simulink library "Garbage"  (R2023a)
settings: Solver=ode45  StartTime=0.0  StopTime=10.0  RelTol=1e-3  EnableMultiTasking=on
undefined symbols (not defined in the file or in its folder): c, ending_column_index, ending_row_index, framebuffer_16x1, framebuffer_2x2, framebuffer_4x4, framebuffer_8x8, HEIGHT, hot, POS_X, POS_Y, starting_column_index, starting_row_index, WIDTH
libraries: Memory_Blocks_Lib x459, simulink x235, simulink_extras x132, dspmtrx3 x116, Bit_Multiplexers_Lib x67, Bit_Demultiplexers_Lib x49, Byte_Multiplexers_Lib x40, Miscellaneous x38, Computer_Library x17, Decoders_Lib x14, commutil2 x12, Arithmetic_Operations_Lib x8
(part 9 of 26)

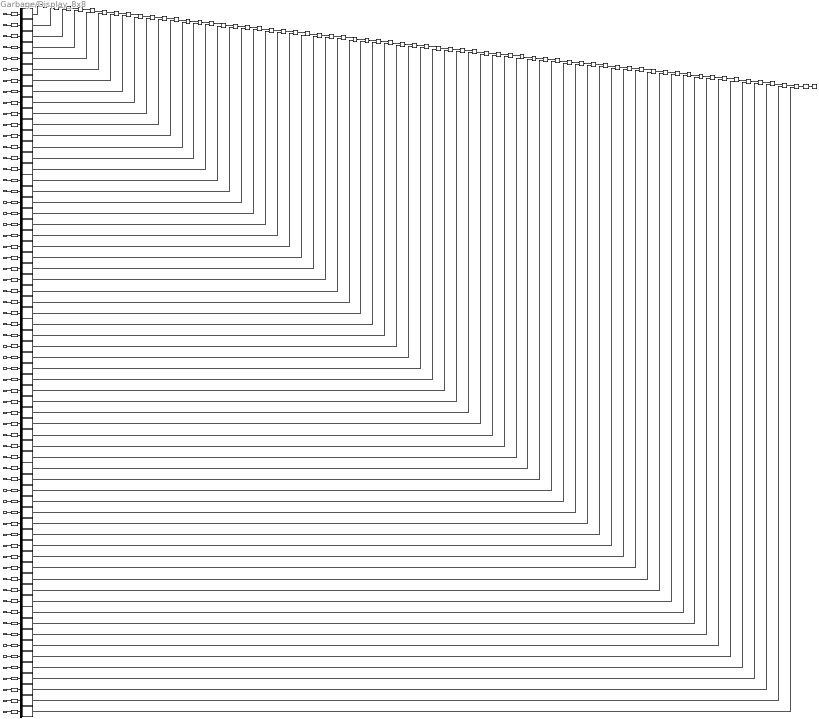
== system Garbage/Display_8x8 ==
  Row1_Col1(8x1B) : Inport
  Row1_Col2(8x1B) : Inport  Port=2
  Row1_Col3(8x1B) : Inport  Port=3
  Row1_Col4(8x1B) : Inport  Port=4
  Row1_Col5(8x1B) : Inport  Port=5
  Row1_Col6(8x1B) : Inport  Port=6
  Row1_Col7(8x1B) : Inport  Port=7
  Row1_Col8(8x1B) : Inport  Port=8
  Row2_Col1(8x1B) : Inport  Port=9
  Row2_Col2(8x1B) : Inport  Port=10
  Row2_Col3(8x1B) : Inport  Port=11
  Row2_Col4(8x1B) : Inport  Port=12
  Row2_Col5(8x1B) : Inport  Port=13
  Row2_Col6(8x1B) : Inport  Port=14
  Row2_Col7(8x1B) : Inport  Port=15
  Row2_Col8(8x1B) : Inport  Port=16
  Row3_Col1(8x1B) : Inport  Port=17
  Row3_Col2(8x1B) : Inport  Port=18
  Row3_Col3(8x1B) : Inport  Port=19
  Row3_Col4(8x1B) : Inport  Port=20
  Row3_Col5(8x1B) : Inport  Port=21
  Row3_Col6(8x1B) : Inport  Port=22
  Row3_Col7(8x1B) : Inport  Port=23
  Row3_Col8(8x1B) : Inport  Port=24
  Row4_Col1(8x1B) : Inport  Port=25
  Row4_Col2(8x1B) : Inport  Port=26
  Row4_Col3(8x1B) : Inport  Port=27
  Row4_Col4(8x1B) : Inport  Port=28
  Row4_Col5(8x1B) : Inport  Port=29
  Row4_Col6(8x1B) : Inport  Port=30
  Row4_Col7(8x1B) : Inport  Port=31
  Row4_Col8(8x1B) : Inport  Port=32
  Row5_Col1(8x1B) : Inport  Port=33
  Row5_Col2(8x1B) : Inport  Port=34
  Row5_Col3(8x1B) : Inport  Port=35
  Row5_Col4(8x1B) : Inport  Port=36
  Row5_Col5(8x1B) : Inport  Port=37
  Row5_Col6(8x1B) : Inport  Port=38
  Row5_Col7(8x1B) : Inport  Port=39
  Row5_Col8(8x1B) : Inport  Port=40
  Row6_Col1(8x1B) : Inport  Port=41
  Row6_Col2(8x1B) : Inport  Port=42
  Row6_Col3(8x1B) : Inport  Port=43
  Row6_Col4(8x1B) : Inport  Port=44
  Row6_Col5(8x1B) : Inport  Port=45
  Row6_Col6(8x1B) : Inport  Port=46
  Row6_Col7(8x1B) : Inport  Port=47
  Row6_Col8(8x1B) : Inport  Port=48
  Row7_Col1(8x1B) : Inport  Port=49
  Row7_Col2(8x1B) : Inport  Port=50
  Row7_Col3(8x1B) : Inport  Port=51
  Row7_Col4(8x1B) : Inport  Port=52
  Row7_Col5(8x1B) : Inport  Port=53
  Row7_Col6(8x1B) : Inport  Port=54
  Row7_Col7(8x1B) : Inport  Port=55
  Row7_Col8(8x1B) : Inport  Port=56
  Row8_Col1(8x1B) : Inport  Port=57
  Row8_Col2(8x1B) : Inport  Port=58
  Row8_Col3(8x1B) : Inport  Port=59
  Row8_Col4(8x1B) : Inport  Port=60
  Row8_Col5(8x1B) : Inport  Port=61
  Row8_Col6(8x1B) : Inport  Port=62
  Row8_Col7(8x1B) : Inport  Port=63
  Row8_Col8(8x1B) : Inport  Port=64
  Constant1 : Constant  Value=framebuffer_8x8  OutDataTypeStr=uint8
  Demux{1..3,20..80} (64 alike) : Demux  Outputs=8
  Integer to Bit Converter{1..3,17,21..80} (64 alike) : simulink/Logic and Bit Operations/Integer to Bit Converter  nbits=8  signedInputValues=Unsigned  bitOrder=MSB first  outDtype=uint8
      settings: LibrarySourceBlock=|
      | commutil2/Integer to Bit
      | Converter
  Matrix Concatenate{1..4,21..80} (64 alike) : Concatenate  NumInputs=8  Mode=Multidimensional array  ConcatenateDimension=2
  Matrix Viewer3 : dspobslib/Matrix Viewer  ImageParams=off  CMapStr=hot(256)  AxisColorbar=on  AxisParams=off  AxisOrigin=Upper left corner  AxisTickMode=Auto  XTickRange=[0 1]  YTickRange=[0 1]  XLabel=X-axis  YLabel=Y-axis  ZLabel=Z-axis  FigPos=[POS_X POS_Y-140 WIDTH HEIGHT]  AxisZoom=on
      settings: YMin=0  YMax=1.0
  Overwrite Values1 : dspmtrx3/Overwrite Values  OverWriteDiag=Submatrix  valFrom2ndIP=Second input port  ConstValue=0  RowSpan=Range of rows  RowStartMode=Index  RowStartIndex=starting_row_index(3)  RowEndMode=Index  RowEndIndex=ending_row_index(3)  ColSpan=Range of columns  ColStartMode=Index  ColStartIndex=starting_column_index(2)  ColEndMode=Index  ColEndIndex=ending_column_index(2)  DiagSpan=Range of elements  DiagStartMode=Index  DiagStartIndex=2  DiagEndMode=Index  DiagEndIndex=4
  Overwrite Values15 : dspmtrx3/Overwrite Values  OverWriteDiag=Submatrix  valFrom2ndIP=Second input port  ConstValue=0  RowSpan=Range of rows  RowStartMode=Index  RowStartIndex=starting_row_index(3)  RowEndMode=Index  RowEndIndex=ending_row_index(3)  ColSpan=Range of columns  ColStartMode=Index  ColStartIndex=starting_column_index(4)  ColEndMode=Index  ColEndIndex=ending_column_index(4)  DiagSpan=Range of elements  DiagStartMode=Index  DiagStartIndex=2  DiagEndMode=Index  DiagEndIndex=4
  Overwrite Values17 : dspmtrx3/Overwrite Values  OverWriteDiag=Submatrix  valFrom2ndIP=Second input port  ConstValue=0  RowSpan=Range of rows  RowStartMode=Index  RowStartIndex=starting_row_index(3)  RowEndMode=Index  RowEndIndex=ending_row_index(3)  ColSpan=Range of columns  ColStartMode=Index  ColStartIndex=starting_column_index(1)  ColEndMode=Index  ColEndIndex=ending_column_index(1)  DiagSpan=Range of elements  DiagStartMode=Index  DiagStartIndex=2  DiagEndMode=Index  DiagEndIndex=4
  Overwrite Values18 : dspmtrx3/Overwrite Values  OverWriteDiag=Submatrix  valFrom2ndIP=Second input port  ConstValue=0  RowSpan=Range of rows  RowStartMode=Index  RowStartIndex=starting_row_index(5)  RowEndMode=Index  RowEndIndex=ending_row_index(5)  ColSpan=Range of columns  ColStartMode=Index  ColStartIndex=starting_column_index(2)  ColEndMode=Index  ColEndIndex=ending_column_index(2)  DiagSpan=Range of elements  DiagStartMode=Index  DiagStartIndex=2  DiagEndMode=Index  DiagEndIndex=4
  Overwrite Values19 : dspmtrx3/Overwrite Values  OverWriteDiag=Submatrix  valFrom2ndIP=Second input port  ConstValue=0  RowSpan=Range of rows  RowStartMode=Index  RowStartIndex=starting_row_index(4)  RowEndMode=Index  RowEndIndex=ending_row_index(4)  ColSpan=Range of columns  ColStartMode=Index  ColStartIndex=starting_column_index(4)  ColEndMode=Index  ColEndIndex=ending_column_index(4)  DiagSpan=Range of elements  DiagStartMode=Index  DiagStartIndex=2  DiagEndMode=Index  DiagEndIndex=4
  Overwrite Values2 : dspmtrx3/Overwrite Values  OverWriteDiag=Submatrix  valFrom2ndIP=Second input port  ConstValue=0  RowSpan=Range of rows  RowStartMode=Index  RowStartIndex=starting_row_index(8)  RowEndMode=Index  RowEndIndex=ending_row_index(8)  ColSpan=Range of columns  ColStartMode=Index  ColStartIndex=starting_column_index(3)  ColEndMode=Index  ColEndIndex=ending_column_index(3)  DiagSpan=Range of elements  DiagStartMode=Index  DiagStartIndex=2  DiagEndMode=Index  DiagEndIndex=4
  Overwrite Values21 : dspmtrx3/Overwrite Values  OverWriteDiag=Submatrix  valFrom2ndIP=Second input port  ConstValue=0  RowSpan=Range of rows  RowStartMode=Index  RowStartIndex=starting_row_index(5)  RowEndMode=Index  RowEndIndex=ending_row_index(5)  ColSpan=Range of columns  ColStartMode=Index  ColStartIndex=starting_column_index(4)  ColEndMode=Index  ColEndIndex=ending_column_index(4)  DiagSpan=Range of elements  DiagStartMode=Index  DiagStartIndex=2  DiagEndMode=Index  DiagEndIndex=4
  Overwrite Values22 : dspmtrx3/Overwrite Values  OverWriteDiag=Submatrix  valFrom2ndIP=Second input port  ConstValue=0  RowSpan=Range of rows  RowStartMode=Index  RowStartIndex=starting_row_index(6)  RowEndMode=Index  RowEndIndex=ending_row_index(6)  ColSpan=Range of columns  ColStartMode=Index  ColStartIndex=starting_column_index(4)  ColEndMode=Index  ColEndIndex=ending_column_index(4)  DiagSpan=Range of elements  DiagStartMode=Index  DiagStartIndex=2  DiagEndMode=Index  DiagEndIndex=4
  Overwrite Values25 : dspmtrx3/Overwrite Values  OverWriteDiag=Submatrix  valFrom2ndIP=Second input port  ConstValue=0  RowSpan=Range of rows  RowStartMode=Index  RowStartIndex=starting_row_index(7)  RowEndMode=Index  RowEndIndex=ending_row_index(7)  ColSpan=Range of columns  ColStartMode=Index  ColStartIndex=starting_column_index(4)  ColEndMode=Index  ColEndIndex=ending_column_index(4)  DiagSpan=Range of elements  DiagStartMode=Index  DiagStartIndex=2  DiagEndMode=Index  DiagEndIndex=4
  Overwrite Values26 : dspmtrx3/Overwrite Values  OverWriteDiag=Submatrix  valFrom2ndIP=Second input port  ConstValue=0  RowSpan=Range of rows  RowStartMode=Index  RowStartIndex=starting_row_index(3)  RowEndMode=Index  RowEndIndex=ending_row_index(3)  ColSpan=Range of columns  ColStartMode=Index  ColStartIndex=starting_column_index(3)  ColEndMode=Index  ColEndIndex=ending_column_index(3)  DiagSpan=Range of elements  DiagStartMode=Index  DiagStartIndex=2  DiagEndMode=Index  DiagEndIndex=4
  Overwrite Values27 : dspmtrx3/Overwrite Values  OverWriteDiag=Submatrix  valFrom2ndIP=Second input port  ConstValue=0  RowSpan=Range of rows  RowStartMode=Index  RowStartIndex=starting_row_index(4)  RowEndMode=Index  RowEndIndex=ending_row_index(4)  ColSpan=Range of columns  ColStartMode=Index  ColStartIndex=starting_column_index(1)  ColEndMode=Index  ColEndIndex=ending_column_index(1)  DiagSpan=Range of elements  DiagStartMode=Index  DiagStartIndex=2  DiagEndMode=Index  DiagEndIndex=4
  Overwrite Values28 : dspmtrx3/Overwrite Values  OverWriteDiag=Submatrix  valFrom2ndIP=Second input port  ConstValue=0  RowSpan=Range of rows  RowStartMode=Index  RowStartIndex=starting_row_index(6)  RowEndMode=Index  RowEndIndex=ending_row_index(6)  ColSpan=Range of columns  ColStartMode=Index  ColStartIndex=starting_column_index(2)  ColEndMode=Index  ColEndIndex=ending_column_index(2)  DiagSpan=Range of elements  DiagStartMode=Index  DiagStartIndex=2  DiagEndMode=Index  DiagEndIndex=4
  Overwrite Values29 : dspmtrx3/Overwrite Values  OverWriteDiag=Submatrix  valFrom2ndIP=Second input port  ConstValue=0  RowSpan=Range of rows  RowStartMode=Index  RowStartIndex=starting_row_index(8)  RowEndMode=Index  RowEndIndex=ending_row_index(8)  ColSpan=Range of columns  ColStartMode=Index  ColStartIndex=starting_column_index(4)  ColEndMode=Index  ColEndIndex=ending_column_index(4)  DiagSpan=Range of elements  DiagStartMode=Index  DiagStartIndex=2  DiagEndMode=Index  DiagEndIndex=4
  Overwrite Values3 : dspmtrx3/Overwrite Values  OverWriteDiag=Submatrix  valFrom2ndIP=Second input port  ConstValue=0  RowSpan=Range of rows  RowStartMode=Index  RowStartIndex=starting_row_index(1)  RowEndMode=Index  RowEndIndex=ending_row_index(1)  ColSpan=Range of columns  ColStartMode=Index  ColStartIndex=starting_column_index(3)  ColEndMode=Index  ColEndIndex=ending_column_index(3)  DiagSpan=Range of elements  DiagStartMode=Index  DiagStartIndex=2  DiagEndMode=Index  DiagEndIndex=4
  Overwrite Values30 : dspmtrx3/Overwrite Values  OverWriteDiag=Submatrix  valFrom2ndIP=Second input port  ConstValue=0  RowSpan=Range of rows  RowStartMode=Index  RowStartIndex=starting_row_index(1)  RowEndMode=Index  RowEndIndex=ending_row_index(1)  ColSpan=Range of columns  ColStartMode=Index  ColStartIndex=starting_column_index(4)  ColEndMode=Index  ColEndIndex=ending_column_index(4)  DiagSpan=Range of elements  DiagStartMode=Index  DiagStartIndex=2  DiagEndMode=Index  DiagEndIndex=4
  Overwrite Values31 : dspmtrx3/Overwrite Values  OverWriteDiag=Submatrix  valFrom2ndIP=Second input port  ConstValue=0  RowSpan=Range of rows  RowStartMode=Index  RowStartIndex=starting_row_index(2)  RowEndMode=Index  RowEndIndex=ending_row_index(2)  ColSpan=Range of columns  ColStartMode=Index  ColStartIndex=starting_column_index(4)  ColEndMode=Index  ColEndIndex=ending_column_index(4)  DiagSpan=Range of elements  DiagStartMode=Index  DiagStartIndex=2  DiagEndMode=Index  DiagEndIndex=4
  Overwrite Values32 : dspmtrx3/Overwrite Values  OverWriteDiag=Submatrix  valFrom2ndIP=Second input port  ConstValue=0  RowSpan=Range of rows  RowStartMode=Index  RowStartIndex=starting_row_index(3)  RowEndMode=Index  RowEndIndex=ending_row_index(3)  ColSpan=Range of columns  ColStartMode=Index  ColStartIndex=starting_column_index(5)  ColEndMode=Index  ColEndIndex=ending_column_index(5)  DiagSpan=Range of elements  DiagStartMode=Index  DiagStartIndex=2  DiagEndMode=Index  DiagEndIndex=4
  Overwrite Values33 : dspmtrx3/Overwrite Values  OverWriteDiag=Submatrix  valFrom2ndIP=Second input port  ConstValue=0  RowSpan=Range of rows  RowStartMode=Index  RowStartIndex=starting_row_index(5)  RowEndMode=Index  RowEndIndex=ending_row_index(5)  ColSpan=Range of columns  ColStartMode=Index  ColStartIndex=starting_column_index(1)  ColEndMode=Index  ColEndIndex=ending_column_index(1)  DiagSpan=Range of elements  DiagStartMode=Index  DiagStartIndex=2  DiagEndMode=Index  DiagEndIndex=4
  Overwrite Values34 : dspmtrx3/Overwrite Values  OverWriteDiag=Submatrix  valFrom2ndIP=Second input port  ConstValue=0  RowSpan=Range of rows  RowStartMode=Index  RowStartIndex=starting_row_index(7)  RowEndMode=Index  RowEndIndex=ending_row_index(7)  ColSpan=Range of columns  ColStartMode=Index  ColStartIndex=starting_column_index(2)  ColEndMode=Index  ColEndIndex=ending_column_index(2)  DiagSpan=Range of elements  DiagStartMode=Index  DiagStartIndex=2  DiagEndMode=Index  DiagEndIndex=4
  Overwrite Values35 : dspmtrx3/Overwrite Values  OverWriteDiag=Submatrix  valFrom2ndIP=Second input port  ConstValue=0  RowSpan=Range of rows  RowStartMode=Index  RowStartIndex=starting_row_index(4)  RowEndMode=Index  RowEndIndex=ending_row_index(4)  ColSpan=Range of columns  ColStartMode=Index  ColStartIndex=starting_column_index(5)  ColEndMode=Index  ColEndIndex=ending_column_index(5)  DiagSpan=Range of elements  DiagStartMode=Index  DiagStartIndex=2  DiagEndMode=Index  DiagEndIndex=4
  Overwrite Values36 : dspmtrx3/Overwrite Values  OverWriteDiag=Submatrix  valFrom2ndIP=Second input port  ConstValue=0  RowSpan=Range of rows  RowStartMode=Index  RowStartIndex=starting_row_index(5)  RowEndMode=Index  RowEndIndex=ending_row_index(5)  ColSpan=Range of columns  ColStartMode=Index  ColStartIndex=starting_column_index(5)  ColEndMode=Index  ColEndIndex=ending_column_index(5)  DiagSpan=Range of elements  DiagStartMode=Index  DiagStartIndex=2  DiagEndMode=Index  DiagEndIndex=4
  Overwrite Values39 : dspmtrx3/Overwrite Values  OverWriteDiag=Submatrix  valFrom2ndIP=Second input port  ConstValue=0  RowSpan=Range of rows  RowStartMode=Index  RowStartIndex=starting_row_index(4)  RowEndMode=Index  RowEndIndex=ending_row_index(4)  ColSpan=Range of columns  ColStartMode=Index  ColStartIndex=starting_column_index(3)  ColEndMode=Index  ColEndIndex=ending_column_index(3)  DiagSpan=Range of elements  DiagStartMode=Index  DiagStartIndex=2  DiagEndMode=Index  DiagEndIndex=4
  Overwrite Values4 : dspmtrx3/Overwrite Values  OverWriteDiag=Submatrix  valFrom2ndIP=Second input port  ConstValue=0  RowSpan=Range of rows  RowStartMode=Index  RowStartIndex=starting_row_index(2)  RowEndMode=Index  RowEndIndex=ending_row_index(2)  ColSpan=Range of columns  ColStartMode=Index  ColStartIndex=starting_column_index(3)  ColEndMode=Index  ColEndIndex=ending_column_index(3)  DiagSpan=Range of elements  DiagStartMode=Index  DiagStartIndex=2  DiagEndMode=Index  DiagEndIndex=4
  Overwrite Values40 : dspmtrx3/Overwrite Values  OverWriteDiag=Submatrix  valFrom2ndIP=Second input port  ConstValue=0  RowSpan=Range of rows  RowStartMode=Index  RowStartIndex=starting_row_index(6)  RowEndMode=Index  RowEndIndex=ending_row_index(6)  ColSpan=Range of columns  ColStartMode=Index  ColStartIndex=starting_column_index(5)  ColEndMode=Index  ColEndIndex=ending_column_index(5)  DiagSpan=Range of elements  DiagStartMode=Index  DiagStartIndex=2  DiagEndMode=Index  DiagEndIndex=4
  Overwrite Values41 : dspmtrx3/Overwrite Values  OverWriteDiag=Submatrix  valFrom2ndIP=Second input port  ConstValue=0  RowSpan=Range of rows  RowStartMode=Index  RowStartIndex=starting_row_index(7)  RowEndMode=Index  RowEndIndex=ending_row_index(7)  ColSpan=Range of columns  ColStartMode=Index  ColStartIndex=starting_column_index(5)  ColEndMode=Index  ColEndIndex=ending_column_index(5)  DiagSpan=Range of elements  DiagStartMode=Index  DiagStartIndex=2  DiagEndMode=Index  DiagEndIndex=4
  Overwrite Values42 : dspmtrx3/Overwrite Values  OverWriteDiag=Submatrix  valFrom2ndIP=Second input port  ConstValue=0  RowSpan=Range of rows  RowStartMode=Index  RowStartIndex=starting_row_index(6)  RowEndMode=Index  RowEndIndex=ending_row_index(6)  ColSpan=Range of columns  ColStartMode=Index  ColStartIndex=starting_column_index(1)  ColEndMode=Index  ColEndIndex=ending_column_index(1)  DiagSpan=Range of elements  DiagStartMode=Index  DiagStartIndex=2  DiagEndMode=Index  DiagEndIndex=4
  Overwrite Values43 : dspmtrx3/Overwrite Values  OverWriteDiag=Submatrix  valFrom2ndIP=Second input port  ConstValue=0  RowSpan=Range of rows  RowStartMode=Index  RowStartIndex=starting_row_index(8)  RowEndMode=Index  RowEndIndex=ending_row_index(8)  ColSpan=Range of columns  ColStartMode=Index  ColStartIndex=starting_column_index(2)  ColEndMode=Index  ColEndIndex=ending_column_index(2)  DiagSpan=Range of elements  DiagStartMode=Index  DiagStartIndex=2  DiagEndMode=Index  DiagEndIndex=4
  Overwrite Values44 : dspmtrx3/Overwrite Values  OverWriteDiag=Submatrix  valFrom2ndIP=Second input port  ConstValue=0  RowSpan=Range of rows  RowStartMode=Index  RowStartIndex=starting_row_index(8)  RowEndMode=Index  RowEndIndex=ending_row_index(8)  ColSpan=Range of columns  ColStartMode=Index  ColStartIndex=starting_column_index(5)  ColEndMode=Index  ColEndIndex=ending_column_index(5)  DiagSpan=Range of elements  DiagStartMode=Index  DiagStartIndex=2  DiagEndMode=Index  DiagEndIndex=4
  Overwrite Values45 : dspmtrx3/Overwrite Values  OverWriteDiag=Submatrix  valFrom2ndIP=Second input port  ConstValue=0  RowSpan=Range of rows  RowStartMode=Index  RowStartIndex=starting_row_index(7)  RowEndMode=Index  RowEndIndex=ending_row_index(7)  ColSpan=Range of columns  ColStartMode=Index  ColStartIndex=starting_column_index(1)  ColEndMode=Index  ColEndIndex=ending_column_index(1)  DiagSpan=Range of elements  DiagStartMode=Index  DiagStartIndex=2  DiagEndMode=Index  DiagEndIndex=4
  Overwrite Values46 : dspmtrx3/Overwrite Values  OverWriteDiag=Submatrix  valFrom2ndIP=Second input port  ConstValue=0  RowSpan=Range of rows  RowStartMode=Index  RowStartIndex=starting_row_index(8)  RowEndMode=Index  RowEndIndex=ending_row_index(8)  ColSpan=Range of columns  ColStartMode=Index  ColStartIndex=starting_column_index(1)  ColEndMode=Index  ColEndIndex=ending_column_index(1)  DiagSpan=Range of elements  DiagStartMode=Index  DiagStartIndex=2  DiagEndMode=Index  DiagEndIndex=4
  Overwrite Values47 : dspmtrx3/Overwrite Values  OverWriteDiag=Submatrix  valFrom2ndIP=Second input port  ConstValue=0  RowSpan=Range of rows  RowStartMode=Index  RowStartIndex=starting_row_index(1)  RowEndMode=Index  RowEndIndex=ending_row_index(1)  ColSpan=Range of columns  ColStartMode=Index  ColStartIndex=starting_column_index(2)  ColEndMode=Index  ColEndIndex=ending_column_index(2)  DiagSpan=Range of elements  DiagStartMode=Index  DiagStartIndex=2  DiagEndMode=Index  DiagEndIndex=4
  Overwrite Values48 : dspmtrx3/Overwrite Values  OverWriteDiag=Submatrix  valFrom2ndIP=Second input port  ConstValue=0  RowSpan=Range of rows  RowStartMode=Index  RowStartIndex=starting_row_index(2)  RowEndMode=Index  RowEndIndex=ending_row_index(2)  ColSpan=Range of columns  ColStartMode=Index  ColStartIndex=starting_column_index(2)  ColEndMode=Index  ColEndIndex=ending_column_index(2)  DiagSpan=Range of elements  DiagStartMode=Index  DiagStartIndex=2  DiagEndMode=Index  DiagEndIndex=4
  Overwrite Values49 : dspmtrx3/Overwrite Values  OverWriteDiag=Submatrix  valFrom2ndIP=Second input port  ConstValue=0  RowSpan=Range of rows  RowStartMode=Index  RowStartIndex=starting_row_index(1)  RowEndMode=Index  RowEndIndex=ending_row_index(1)  ColSpan=Range of columns  ColStartMode=Index  ColStartIndex=starting_column_index(5)  ColEndMode=Index  ColEndIndex=ending_column_index(5)  DiagSpan=Range of elements  DiagStartMode=Index  DiagStartIndex=2  DiagEndMode=Index  DiagEndIndex=4
  Overwrite Values50 : dspmtrx3/Overwrite Values  OverWriteDiag=Submatrix  valFrom2ndIP=Second input port  ConstValue=0  RowSpan=Range of rows  RowStartMode=Index  RowStartIndex=starting_row_index(5)  RowEndMode=Index  RowEndIndex=ending_row_index(5)  ColSpan=Range of columns  ColStartMode=Index  ColStartIndex=starting_column_index(3)  ColEndMode=Index  ColEndIndex=ending_column_index(3)  DiagSpan=Range of elements  DiagStartMode=Index  DiagStartIndex=2  DiagEndMode=Index  DiagEndIndex=4
  Overwrite Values51 : dspmtrx3/Overwrite Values  OverWriteDiag=Submatrix  valFrom2ndIP=Second input port  ConstValue=0  RowSpan=Range of rows  RowStartMode=Index  RowStartIndex=starting_row_index(2)  RowEndMode=Index  RowEndIndex=ending_row_index(2)  ColSpan=Range of columns  ColStartMode=Index  ColStartIndex=starting_column_index(5)  ColEndMode=Index  ColEndIndex=ending_column_index(5)  DiagSpan=Range of elements  DiagStartMode=Index  DiagStartIndex=2  DiagEndMode=Index  DiagEndIndex=4
  Overwrite Values52 : dspmtrx3/Overwrite Values  OverWriteDiag=Submatrix  valFrom2ndIP=Second input port  ConstValue=0  RowSpan=Range of rows  RowStartMode=Index  RowStartIndex=starting_row_index(3)  RowEndMode=Index  RowEndIndex=ending_row_index(3)  ColSpan=Range of columns  ColStartMode=Index  ColStartIndex=starting_column_index(6)  ColEndMode=Index  ColEndIndex=ending_column_index(6)  DiagSpan=Range of elements  DiagStartMode=Index  DiagStartIndex=2  DiagEndMode=Index  DiagEndIndex=4
  Overwrite Values53 : dspmtrx3/Overwrite Values  OverWriteDiag=Submatrix  valFrom2ndIP=Second input port  ConstValue=0  RowSpan=Range of rows  RowStartMode=Index  RowStartIndex=starting_row_index(4)  RowEndMode=Index  RowEndIndex=ending_row_index(4)  ColSpan=Range of columns  ColStartMode=Index  ColStartIndex=starting_column_index(6)  ColEndMode=Index  ColEndIndex=ending_column_index(6)  DiagSpan=Range of elements  DiagStartMode=Index  DiagStartIndex=2  DiagEndMode=Index  DiagEndIndex=4
  Overwrite Values54 : dspmtrx3/Overwrite Values  OverWriteDiag=Submatrix  valFrom2ndIP=Second input port  ConstValue=0  RowSpan=Range of rows  RowStartMode=Index  RowStartIndex=starting_row_index(5)  RowEndMode=Index  RowEndIndex=ending_row_index(5)  ColSpan=Range of columns  ColStartMode=Index  ColStartIndex=starting_column_index(6)  ColEndMode=Index  ColEndIndex=ending_column_index(6)  DiagSpan=Range of elements  DiagStartMode=Index  DiagStartIndex=2  DiagEndMode=Index  DiagEndIndex=4
  Overwrite Values55 : dspmtrx3/Overwrite Values  OverWriteDiag=Submatrix  valFrom2ndIP=Second input port  ConstValue=0  RowSpan=Range of rows  RowStartMode=Index  RowStartIndex=starting_row_index(6)  RowEndMode=Index  RowEndIndex=ending_row_index(6)  ColSpan=Range of columns  ColStartMode=Index  ColStartIndex=starting_column_index(6)  ColEndMode=Index  ColEndIndex=ending_column_index(6)  DiagSpan=Range of elements  DiagStartMode=Index  DiagStartIndex=2  DiagEndMode=Index  DiagEndIndex=4
  Overwrite Values56 : dspmtrx3/Overwrite Values  OverWriteDiag=Submatrix  valFrom2ndIP=Second input port  ConstValue=0  RowSpan=Range of rows  RowStartMode=Index  RowStartIndex=starting_row_index(7)  RowEndMode=Index  RowEndIndex=ending_row_index(7)  ColSpan=Range of columns  ColStartMode=Index  ColStartIndex=starting_column_index(6)  ColEndMode=Index  ColEndIndex=ending_column_index(6)  DiagSpan=Range of elements  DiagStartMode=Index  DiagStartIndex=2  DiagEndMode=Index  DiagEndIndex=4
  Overwrite Values57 : dspmtrx3/Overwrite Values  OverWriteDiag=Submatrix  valFrom2ndIP=Second input port  ConstValue=0  RowSpan=Range of rows  RowStartMode=Index  RowStartIndex=starting_row_index(8)  RowEndMode=Index  RowEndIndex=ending_row_index(8)  ColSpan=Range of columns  ColStartMode=Index  ColStartIndex=starting_column_index(6)  ColEndMode=Index  ColEndIndex=ending_column_index(6)  DiagSpan=Range of elements  DiagStartMode=Index  DiagStartIndex=2  DiagEndMode=Index  DiagEndIndex=4
  Overwrite Values58 : dspmtrx3/Overwrite Values  OverWriteDiag=Submatrix  valFrom2ndIP=Second input port  ConstValue=0  RowSpan=Range of rows  RowStartMode=Index  RowStartIndex=starting_row_index(1)  RowEndMode=Index  RowEndIndex=ending_row_index(1)  ColSpan=Range of columns  ColStartMode=Index  ColStartIndex=starting_column_index(6)  ColEndMode=Index  ColEndIndex=ending_column_index(6)  DiagSpan=Range of elements  DiagStartMode=Index  DiagStartIndex=2  DiagEndMode=Index  DiagEndIndex=4
  Overwrite Values59 : dspmtrx3/Overwrite Values  OverWriteDiag=Submatrix  valFrom2ndIP=Second input port  ConstValue=0  RowSpan=Range of rows  RowStartMode=Index  RowStartIndex=starting_row_index(2)  RowEndMode=Index  RowEndIndex=ending_row_index(2)  ColSpan=Range of columns  ColStartMode=Index  ColStartIndex=starting_column_index(6)  ColEndMode=Index  ColEndIndex=ending_column_index(6)  DiagSpan=Range of elements  DiagStartMode=Index  DiagStartIndex=2  DiagEndMode=Index  DiagEndIndex=4
  Overwrite Values60 : dspmtrx3/Overwrite Values  OverWriteDiag=Submatrix  valFrom2ndIP=Second input port  ConstValue=0  RowSpan=Range of rows  RowStartMode=Index  RowStartIndex=starting_row_index(3)  RowEndMode=Index  RowEndIndex=ending_row_index(3)  ColSpan=Range of columns  ColStartMode=Index  ColStartIndex=starting_column_index(7)  ColEndMode=Index  ColEndIndex=ending_column_index(7)  DiagSpan=Range of elements  DiagStartMode=Index  DiagStartIndex=2  DiagEndMode=Index  DiagEndIndex=4
  Overwrite Values61 : dspmtrx3/Overwrite Values  OverWriteDiag=Submatrix  valFrom2ndIP=Second input port  ConstValue=0  RowSpan=Range of rows  RowStartMode=Index  RowStartIndex=starting_row_index(6)  RowEndMode=Index  RowEndIndex=ending_row_index(6)  ColSpan=Range of columns  ColStartMode=Index  ColStartIndex=starting_column_index(3)  ColEndMode=Index  ColEndIndex=ending_column_index(3)  DiagSpan=Range of elements  DiagStartMode=Index  DiagStartIndex=2  DiagEndMode=Index  DiagEndIndex=4
  Overwrite Values62 : dspmtrx3/Overwrite Values  OverWriteDiag=Submatrix  valFrom2ndIP=Second input port  ConstValue=0  RowSpan=Range of rows  RowStartMode=Index  RowStartIndex=starting_row_index(4)  RowEndMode=Index  RowEndIndex=ending_row_index(4)  ColSpan=Range of columns  ColStartMode=Index  ColStartIndex=starting_column_index(7)  ColEndMode=Index  ColEndIndex=ending_column_index(7)  DiagSpan=Range of elements  DiagStartMode=Index  DiagStartIndex=2  DiagEndMode=Index  DiagEndIndex=4
  Overwrite Values63 : dspmtrx3/Overwrite Values  OverWriteDiag=Submatrix  valFrom2ndIP=Second input port  ConstValue=0  RowSpan=Range of rows  RowStartMode=Index  RowStartIndex=starting_row_index(5)  RowEndMode=Index  RowEndIndex=ending_row_index(5)  ColSpan=Range of columns  ColStartMode=Index  ColStartIndex=starting_column_index(7)  ColEndMode=Index  ColEndIndex=ending_column_index(7)  DiagSpan=Range of elements  DiagStartMode=Index  DiagStartIndex=2  DiagEndMode=Index  DiagEndIndex=4
  Overwrite Values64 : dspmtrx3/Overwrite Values  OverWriteDiag=Submatrix  valFrom2ndIP=Second input port  ConstValue=0  RowSpan=Range of rows  RowStartMode=Index  RowStartIndex=starting_row_index(6)  RowEndMode=Index  RowEndIndex=ending_row_index(6)  ColSpan=Range of columns  ColStartMode=Index  ColStartIndex=starting_column_index(7)  ColEndMode=Index  ColEndIndex=ending_column_index(7)  DiagSpan=Range of elements  DiagStartMode=Index  DiagStartIndex=2  DiagEndMode=Index  DiagEndIndex=4
  Overwrite Values65 : dspmtrx3/Overwrite Values  OverWriteDiag=Submatrix  valFrom2ndIP=Second input port  ConstValue=0  RowSpan=Range of rows  RowStartMode=Index  RowStartIndex=starting_row_index(7)  RowEndMode=Index  RowEndIndex=ending_row_index(7)  ColSpan=Range of columns  ColStartMode=Index  ColStartIndex=starting_column_index(7)  ColEndMode=Index  ColEndIndex=ending_column_index(7)  DiagSpan=Range of elements  DiagStartMode=Index  DiagStartIndex=2  DiagEndMode=Index  DiagEndIndex=4
  Overwrite Values66 : dspmtrx3/Overwrite Values  OverWriteDiag=Submatrix  valFrom2ndIP=Second input port  ConstValue=0  RowSpan=Range of rows  RowStartMode=Index  RowStartIndex=starting_row_index(8)  RowEndMode=Index  RowEndIndex=ending_row_index(8)  ColSpan=Range of columns  ColStartMode=Index  ColStartIndex=starting_column_index(7)  ColEndMode=Index  ColEndIndex=ending_column_index(7)  DiagSpan=Range of elements  DiagStartMode=Index  DiagStartIndex=2  DiagEndMode=Index  DiagEndIndex=4
  Overwrite Values67 : dspmtrx3/Overwrite Values  OverWriteDiag=Submatrix  valFrom2ndIP=Second input port  ConstValue=0  RowSpan=Range of rows  RowStartMode=Index  RowStartIndex=starting_row_index(1)  RowEndMode=Index  RowEndIndex=ending_row_index(1)  ColSpan=Range of columns  ColStartMode=Index  ColStartIndex=starting_column_index(7)  ColEndMode=Index  ColEndIndex=ending_column_index(7)  DiagSpan=Range of elements  DiagStartMode=Index  DiagStartIndex=2  DiagEndMode=Index  DiagEndIndex=4
  Overwrite Values68 : dspmtrx3/Overwrite Values  OverWriteDiag=Submatrix  valFrom2ndIP=Second input port  ConstValue=0  RowSpan=Range of rows  RowStartMode=Index  RowStartIndex=starting_row_index(2)  RowEndMode=Index  RowEndIndex=ending_row_index(2)  ColSpan=Range of columns  ColStartMode=Index  ColStartIndex=starting_column_index(7)  ColEndMode=Index  ColEndIndex=ending_column_index(7)  DiagSpan=Range of elements  DiagStartMode=Index  DiagStartIndex=2  DiagEndMode=Index  DiagEndIndex=4
  Overwrite Values69 : dspmtrx3/Overwrite Values  OverWriteDiag=Submatrix  valFrom2ndIP=Second input port  ConstValue=0  RowSpan=Range of rows  RowStartMode=Index  RowStartIndex=starting_row_index(3)  RowEndMode=Index  RowEndIndex=ending_row_index(3)  ColSpan=Range of columns  ColStartMode=Index  ColStartIndex=starting_column_index(8)  ColEndMode=Index  ColEndIndex=ending_column_index(8)  DiagSpan=Range of elements  DiagStartMode=Index  DiagStartIndex=2  DiagEndMode=Index  DiagEndIndex=4
  Overwrite Values70 : dspmtrx3/Overwrite Values  OverWriteDiag=Submatrix  valFrom2ndIP=Second input port  ConstValue=0  RowSpan=Range of rows  RowStartMode=Index  RowStartIndex=starting_row_index(4)  RowEndMode=Index  RowEndIndex=ending_row_index(4)  ColSpan=Range of columns  ColStartMode=Index  ColStartIndex=starting_column_index(8)  ColEndMode=Index  ColEndIndex=ending_column_index(8)  DiagSpan=Range of elements  DiagStartMode=Index  DiagStartIndex=2  DiagEndMode=Index  DiagEndIndex=4
  Overwrite Values71 : dspmtrx3/Overwrite Values  OverWriteDiag=Submatrix  valFrom2ndIP=Second input port  ConstValue=0  RowSpan=Range of rows  RowStartMode=Index  RowStartIndex=starting_row_index(5)  RowEndMode=Index  RowEndIndex=ending_row_index(5)  ColSpan=Range of columns  ColStartMode=Index  ColStartIndex=starting_column_index(8)  ColEndMode=Index  ColEndIndex=ending_column_index(8)  DiagSpan=Range of elements  DiagStartMode=Index  DiagStartIndex=2  DiagEndMode=Index  DiagEndIndex=4
  Overwrite Values72 : dspmtrx3/Overwrite Values  OverWriteDiag=Submatrix  valFrom2ndIP=Second input port  ConstValue=0  RowSpan=Range of rows  RowStartMode=Index  RowStartIndex=starting_row_index(7)  RowEndMode=Index  RowEndIndex=ending_row_index(7)  ColSpan=Range of columns  ColStartMode=Index  ColStartIndex=starting_column_index(3)  ColEndMode=Index  ColEndIndex=ending_column_index(3)  DiagSpan=Range of elements  DiagStartMode=Index  DiagStartIndex=2  DiagEndMode=Index  DiagEndIndex=4
  Overwrite Values73 : dspmtrx3/Overwrite Values  OverWriteDiag=Submatrix  valFrom2ndIP=Second input port  ConstValue=0  RowSpan=Range of rows  RowStartMode=Index  RowStartIndex=starting_row_index(6)  RowEndMode=Index  RowEndIndex=ending_row_index(6)  ColSpan=Range of columns  ColStartMode=Index  ColStartIndex=starting_column_index(8)  ColEndMode=Index  ColEndIndex=ending_column_index(8)  DiagSpan=Range of elements  DiagStartMode=Index  DiagStartIndex=2  DiagEndMode=Index  DiagEndIndex=4
  Overwrite Values74 : dspmtrx3/Overwrite Values  OverWriteDiag=Submatrix  valFrom2ndIP=Second input port  ConstValue=0  RowSpan=Range of rows  RowStartMode=Index  RowStartIndex=starting_row_index(7)  RowEndMode=Index  RowEndIndex=ending_row_index(7)  ColSpan=Range of columns  ColStartMode=Index  ColStartIndex=starting_column_index(8)  ColEndMode=Index  ColEndIndex=ending_column_index(8)  DiagSpan=Range of elements  DiagStartMode=Index  DiagStartIndex=2  DiagEndMode=Index  DiagEndIndex=4
  Overwrite Values75 : dspmtrx3/Overwrite Values  OverWriteDiag=Submatrix  valFrom2ndIP=Second input port  ConstValue=0  RowSpan=Range of rows  RowStartMode=Index  RowStartIndex=starting_row_index(8)  RowEndMode=Index  RowEndIndex=ending_row_index(8)  ColSpan=Range of columns  ColStartMode=Index  ColStartIndex=starting_column_index(8)  ColEndMode=Index  ColEndIndex=ending_column_index(8)  DiagSpan=Range of elements  DiagStartMode=Index  DiagStartIndex=2  DiagEndMode=Index  DiagEndIndex=4